simulink model "PrkgClimaActvMgr"  (R2022b)
settings: Solver=FixedStepDiscrete  StartTime=0.0  StopTime=10.0  FixedStep=0.2  RelTol=1e-3
(part 3 of 4)

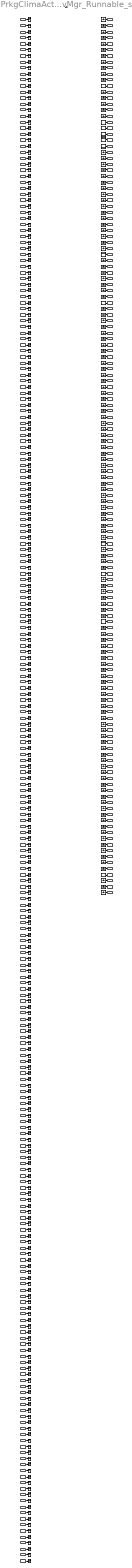
== system PrkgClimaActvMgr/PrkgClimaActvMgr_Runnable_sys ==
  ActvRqrdForPostPrkgClima_ActvRqrdForPostPrkgClima_read : Inport
  CarTiIntForClima_CarTiIntForClima_read : Inport  Port=2
  CdsClimaOnReqfromExsrc_CdsClimaOnReqfromExsrc_read : Inport  Port=3
  ClimaCfgForClimaCtrlMan1_ClimaCfgForClimaCtrlMan1_read : Inport  Port=4
  ClimaCfgForCmptmtClng_ClimaCfgForCmptmtClng_read : Inport  Port=5
  ClimaCfgForFoldCSD_ClimaCfgForFoldCSD_read : Inport  Port=6
  ClimaCfgForNrOfTSetg1_ClimaCfgForNrOfTSetg1_read : Inport  Port=7
  ClimaCfgForPreClng1_ClimaCfgForPreClng1_read : Inport  Port=8
  ClimaCfgForSeatHeatgForRowFirstPrsnt1_ClimaCfgForSeatHeatgForRowFirstPrsnt1_read : Inport  Port=9
  ClimaCfgForSeatHeatgForRowSec_ClimaCfgForSeatHeatgForRowSec_read : Inport  Port=10
  ClimaCfgForSeatVentnForRowFirst_ClimaCfgForSeatVentnForRowFirst_read : Inport  Port=11
  ClimaCfgForSeatVentnForRowSec_ClimaCfgForSeatVentnForRowSec_read : Inport  Port=12
  ClimaCfgForSteerWhlHeatgPrsnt1_ClimaCfgForSteerWhlHeatgPrsnt1_read : Inport  Port=13
  ClimaCfgForSteerWhlLeSide1_ClimaCfgForSteerWhlLeSide1_read : Inport  Port=14
  ClimaCfgForVehElec1_ClimaCfgForVehElec1_read : Inport  Port=15
  ClimaCfgForVehHyb1_ClimaCfgForVehHyb1_read : Inport  Port=16
  ClimaCfgForWindHeatrPrsnt1_ClimaCfgForWindHeatrPrsnt1_read : Inport  Port=17
  ClimaOvrHeatProWarnIni_ClimaOvrHeatProWarnIni_read : Inport  Port=18
  ClimaOvrHeatReqFromExrsc_ClimaOvrHeatReqFromExrsc_read : Inport  Port=19
  ClimaOvrHeatStrtReq_ClimaOvrHeatStrtReq_read : Inport  Port=20
  CmptmtClsd_CmptmtClsd_read : Inport  Port=21
  CSDUsgChgeOffReq_CSDUsgChgeOffReq_read : Inport  Port=22
  CSDUsgChgeOnReq_CSDUsgChgeOnReq_read : Inport  Port=23
  DefrstHeatgForPrkgClimaEnad_DefrstHeatgForPrkgClimaEnad_read : Inport  Port=24
  DoorOpenStsForClima_DoorOpenStsForClima_read : Inport  Port=25
  EgyAllwdForPrkgClima_EgyAllwdForPrkgClima_read : Inport  Port=26
  EgyPrioForPrkgClima_EgyPrioForPrkgClima_read : Inport  Port=27
  ErsCmdByKeyStrtReq_ErsCmdByKeyStrtReq_read : Inport  Port=28
  ErsRunTimelStrtReq_ErsRunTimelStrtReq_read : Inport  Port=29
  FacForRtForPrkgClima_FacForRtForPrkgClima_read : Inport  Port=30
  FrntHvacBlowerSts_FrntHvacBlowerSts_read : Inport  Port=31
  HeatrTypForPrkgClima_HeatrTypForPrkgClima_read : Inport  Port=32
  HeatUpRqrdForPrkgClima_HeatUpRqrdForPrkgClima_read : Inport  Port=33
  HMIExtVentFanVolReqStrtReq_HMIExtVentFanVolReqStrtReq_read : Inport  Port=34
  HMIExtVentonoffReqStrtReq_HMIExtVentonoffReqStrtReq_read : Inport  Port=35
  HMIExtVentTSpReqStrtReq_HMIExtVentTSpReqStrtReq_read : Inport  Port=36
  HMIExtVentTStsReqStrtReq_HMIExtVentTStsReqStrtReq_read : Inport  Port=37
  HvBattForPrkgClima_HvBattForPrkgClima_read : Inport  Port=38
  HvBattSoc_HvBattSoc_read : Inport  Port=39
  HvSysCnctWoU_HvSysCnctWoU_read : Inport  Port=40
  HvSysWoU_HvSysWoU_read : Inport  Port=41
  HvSysRlySts_HvSysRlySts_read : Inport  Port=42
  IntCo2MontrStrtReq_IntCo2MontrStrtReq_read : Inport  Port=43
  LockgCenSts_Ngcc_LockgCenSts_read : Inport  Port=44
  MemReadForPrkgClimaActvMgr_MemReadForPrkgClimaActvMgr_read : Inport  Port=45
  MemReqStsForPrkgClima_MemReqStsForPrkgClima_read : Inport  Port=46
  OffForTLoForPostCdng_OffForTLoForPostCdng_read : Inport  Port=47
  OffRqrdForPrkgClima_OffRqrdForPrkgClima_read : Inport  Port=48
  PrePrkgClimaNotAvlForHvSysWoU_PrePrkgClimaNotAvlForHvSysWoU_read : Inport  Port=49
  PrkgCDSAvl_PrkgCDSAvl_read : Inport  Port=50
  PrkgClimaAllwdForBattULo_PrkgClimaAllwdForBattULo_read : Inport  Port=51
  PrkgClimaAllwdForSwtHeatrTyp_PrkgClimaAllwdForSwtHeatrTyp_read : Inport  Port=52
  PrkgHeatgActv_PrkgHeatgActv_read : Inport  Port=53
  PwrAllwdForHvacFanFrnt_PwrAllwdForHvacFanFrnt_read : Inport  Port=54
  PwrCnsDesForHvacFanFrnt_PwrCnsDesForHvacFanFrnt_read : Inport  Port=55
  RemClimaallExsrcReq_RemClimaallExsrcReq_read : Inport  Port=56
  RemClimaEgyExsrcReq_RemClimaEgyExsrcReq_read : Inport  Port=57
  RemClimaTmrStrtReq_RemClimaTmrStrtReq_read : Inport  Port=58
  RemStrtExtnTiStrtReq_RemStrtExtnTiStrtReq_read : Inport  Port=59
  RemStrtHvCtrlStrtReq_RemStrtHvCtrlStrtReq_read : Inport  Port=60
  RemVentReqStrtReq_RemVentReqStrtReq_read : Inport  Port=61
  RtCmftForImdtPrePrkgClima_RtCmftForImdtPrePrkgClima_read : Inport  Port=62
  RtCmftForPostPrkgClima_RtCmftForPostPrkgClima_read : Inport  Port=63
  RtCmftForPreClng_RtCmftForPreClng_read : Inport  Port=64
  RtSdlForPrkgClima_RtSdlForPrkgClima_read : Inport  Port=65
  SeatHeatForPrkgClimaEnad_SeatHeatForPrkgClimaEnad_read : Inport  Port=66
  SteerWhlHeatgForPrkgClimaEnad_SteerWhlHeatgForPrkgClimaEnad_read : Inport  Port=67
  StrtRemSts1WdStrtRemSts_Ngcc_StrtRemSts1WdStrtRemSts_read : Inport  Port=68
  StrtRqrdForDirForPrePrkgClima_StrtRqrdForDirForPrePrkgClima_read : Inport  Port=69
  StrtRqrdForPreClng_StrtRqrdForPreClng_read : Inport  Port=70
  StrtRqrdForTmrForPrePrkgClima_StrtRqrdForTmrForPrePrkgClima_read : Inport  Port=71
  SuspStrtAllwdForPrkgClima_SuspStrtAllwdForPrkgClima_read : Inport  Port=72
  TelmBookClimaReqHmiCmptmtTSpSpclStrtReq_TelmBookClimaReqHmiCmptmtTSpSpclStrtReq_read : Inport  Port=73
  TelmBookClimaReqOnOffNoReqStrtReq_TelmBookClimaReqOnOffNoReqStrtReq_read : Inport  Port=74
  TelmBookClimaReqTempRangeStrtReq_TelmBookClimaReqTempRangeStrtReq_read : Inport  Port=75
  TelmBookClimaTmrStrtReq_TelmBookClimaTmrStrtReq_read : Inport  Port=76
  TelmBookSeatHeatClimalReqDrvHeatClimaLvlStrtReq_TelmBookSeatHeatClimalReqDrvHeatClimaLvlStrtReq_read : Inport  Port=77
  TelmBookSeatHeatClimalReqPassHeatClimaLvlStrtReq_TelmBookSeatHeatClimalReqPassHeatClimaLvlStrtReq_read : Inport  Port=78
  TelmBookSeatHeatClimalReqSecLeHeatClimaLvlStrtReq_TelmBookSeatHeatClimalReqSecLeHeatClimaLvlStrtReq_read : Inport  Port=79
  TelmBookSeatHeatClimalReqSecRiHeatClimaLvlStrtReq_TelmBookSeatHeatClimalReqSecRiHeatClimaLvlStrtReq_read : Inport  Port=80
  TelmBookSeatHeatClimalReqThrdLeHeatClimaLvlStrtReq_TelmBookSeatHeatClimalReqThrdLeHeatClimaLvlStrtReq_read : Inport  Port=81
  TelmBookSeatHeatClimalReqThrdRiHeatClimaLvlStrtReq_TelmBookSeatHeatClimalReqThrdRiHeatClimaLvlStrtReq_read : Inport  Port=82
  TelmBookSeatVentnClimalReqDrvVentnClimaLvlStrtReq_TelmBookSeatVentnClimalReqDrvVentnClimaLvlStrtReq_read : Inport  Port=83
  TelmBookSeatVentnClimalReqPassVentnClimaLvlStrtReq_TelmBookSeatVentnClimalReqPassVentnClimaLvlStrtReq_read : Inport  Port=84
  TelmBookSeatVentnClimalReqSecLeVentnClimaLvlStrtReq_TelmBookSeatVentnClimalReqSecLeVentnClimaLvlStrtReq_read : Inport  Port=85
  TelmBookSeatVentnClimalReqSecRiVentnClimaLvlStrtReq_TelmBookSeatVentnClimalReqSecRiVentnClimaLvlStrtReq_read : Inport  Port=86
  TelmBookSeatVentnClimalReqThrdLeVentnClimaLvlStrtReq_TelmBookSeatVentnClimalReqThrdLeVentnClimaLvlStrtReq_read : Inport  Port=87
  TelmBookSeatVentnClimalReqThrdRiVentnClimaLvlStrtReq_TelmBookSeatVentnClimalReqThrdRiVentnClimaLvlStrtReq_read : Inport  Port=88
  TelmBookSteerWhlHeatClimalReqStrtReq_TelmBookSteerWhlHeatClimalReqStrtReq_read : Inport  Port=89
  TelmClimaExtVentnLvlStrtReq_TelmClimaExtVentnLvlStrtReq_read : Inport  Port=90
  TelmClimaExtVentnReqStrtReq_TelmClimaExtVentnReqStrtReq_read : Inport  Port=91
  TelmClimaExtVentnTempRangeStrtReq_TelmClimaExtVentnTempRangeStrtReq_read : Inport  Port=92
  TelmClimaExtVentnTSpSpclStrtReq_TelmClimaExtVentnTSpSpclStrtReq_read : Inport  Port=93
  UpdRtTotForTmrForPrePrkgClima_UpdRtTotForTmrForPrePrkgClima_read : Inport  Port=94
  VehModMngtGlbSafe1_Ngcc_VehModMngtGlbSafe1_read : Inport  Port=95
  VFCBodyPreClimatisationHmiHumCtrl_VFCBodyPreClimatisationHmiHumCtrl_read : Inport  Port=96
  VFCHmiClimaCtrlAct_VFCHmiClimaCtrlAct_read : Inport  Port=97
  VFCPrkDrvgClimabyFragAct_VFCPrkDrvgClimabyFragAct_read : Inport  Port=98
  WarnForEgyAllwdLimd_WarnForEgyAllwdLimd_read : Inport  Port=99
  WarnForHybWoU_WarnForHybWoU_read : Inport  Port=100
  WarnForPreCdngFctNotAvl_WarnForPreCdngFctNotAvl_read : Inport  Port=101
  WarnForPrkgClimaNotAllwd_WarnForPrkgClimaNotAllwd_read : Inport  Port=102
  WindIceDetdFrnt_Ngcc_WindIceDetdFrnt_read : Inport  Port=103
  WindIceDetdRe_Ngcc_WindIceDetdRe_read : Inport  Port=104
  AirCdnAvl_AirCdnAvl_read : Inport  Port=105
  AmbTFildForClimaNgcc_AmbTFildForClima_read : Inport  Port=106
  OffRqrdForRemClima_OffRqrdForRemClima_read : Inport  Port=107
  RemClimaTempStrtReq_RemClimaTempStrtReq_read : Inport  Port=108
  VFCAutDemstOperSts_VFCAutDemstOperSts_read : Inport  Port=109
  VFCPrkDrvgClimabyPM25_VFCPrkDrvgClimabyPM25_read : Inport  Port=110
  AirCdnAvlRe_AirCdnAvlRe_read : Inport  Port=111
  AirSrcStsFbNgcc_AirSrcStsFbNgcc_read : Inport  Port=112
  BookTrvlClimaActvReqfromExsrc_BookTrvlClimaActvReqfromExsrc_read : Inport  Port=113
  CCMWakeupFunction_CCMWakeupFunction_read : Inport  Port=114
  ClimaCfgFor3RDROWSEATHEAT_ClimaCfgFor3RDROWSEATHEAT_read : Inport  Port=115
  ClimaCfgFor3RDROWSEATVENTILATED_ClimaCfgFor3RDROWSEATVENTILATED_read : Inport  Port=116
  ClimaCfgForAirMtrlSnsrPrsnt1_ClimaCfgForAirMtrlSnsrPrsnt1_read : Inport  Port=117
  ClimaCfgForBRAND_ClimaCfgForBRAND_read : Inport  Port=118
  ClimaCfgForDrvrSeatVentWthSmf2_ClimaCfgForDrvrSeatVentWthSmf2_read : Inport  Port=119
  ClimaCfgForErev1_ClimaCfgForErev1_read : Inport  Port=120
  ClimaCfgForHvacRePrsnt1_ClimaCfgForHvacRePrsnt1_read : Inport  Port=121
  ClimaCfgForHvacTyp_ClimaCfgForHvacTyp_read : Inport  Port=122
  ClimaCfgForLifeDetection_ClimaCfgForLifeDetection_read : Inport  Port=123
  ClimaCfgForMARKET_ClimaCfgForMARKET_read : Inport  Port=124
  ClimaCfgForPassSeatVentWthSmf2_ClimaCfgForPassSeatVentWthSmf2_read : Inport  Port=125
  ClimaCfgForPrpsnTyp_ClimaCfgForPrpsnTyp_read : Inport  Port=126
  ClimaCfgForRoofHatch_ClimaCfgForRoofHatch_read : Inport  Port=127
  ClimaCfgForVehTyp_ClimaCfgForVehTyp_read : Inport  Port=128
  ClimaCfgReqVersionReplace_ClimaCfgReqVersionReplace_read : Inport  Port=129
  CmptmtClngActv_CmptmtClngActv_read : Inport  Port=130
  CmptmtOpenStsForClima_CmptmtOpenStsForClima_read : Inport  Port=131
  DoorStsWithOutTrForClima_DoorStsWithOutTrForClima_read : Inport  Port=132
  DrvrSeatHeatgLvlSts_DrvrSeatHeatgLvlSts_read : Inport  Port=133
  DrvrSeatHeatgLvlSts_DrvrSeatHeatgLvlSts_IsUpdated_read : Inport  Port=134
  DrvrSeatVentnLvlSts_DrvrSeatVentnLvlSts_read : Inport  Port=135
  DrvrSeatVentnLvlSts_DrvrSeatVentnLvlSts_IsUpdated_read : Inport  Port=136
  DrvrSeatVentnLvlSts2Ngcc_DrvrSeatVentnLvlSts2Ngcc_read : Inport  Port=137
  EngSt1WdSts_Ngcc_EngSt1WdSts_read : Inport  Port=138
  ErsCmdByKeyFromExsrc_ErsCmdByKeyFromExsrc_read : Inport  Port=139
  GearLvrIndcn_GearLvrIndcn_read : Inport  Port=140
  GearLvrIndcn_GearLvrIndcn_IsUpdated_read : Inport  Port=141
  HighTempCleanHmiReqNgcc_HighTempCleanHmiReqNgcc_read : Inport  Port=142
  HMIAirCdnrAutDrySetNgcc_HMIAirCdnrAutDrySetNgcc_read : Inport  Port=143
  HMIAirCdnrAutDrySetNgcc_HMIAirCdnrAutDrySetNgcc_IsUpdated_read : Inport  Port=144
  HmiCmptmtTSp_HmiCmptmtTSp_read : Inport  Port=145
  HMILBRACStrtSetNgcc_HMILBRACStrtSetNgcc_read : Inport  Port=146
  HmiParkVentSetNgcc_HmiParkVentSetNgcc_read : Inport  Port=147
  HmiParkVentTmrSetNgcc_HmiParkVentTmrSetNgcc_read : Inport  Port=148
  HvBattMntnActv_HvBattMntnActv_read : Inport  Port=149
  HvCooltHeatrStsSig_HvCooltHeatrStsSig_read : Inport  Port=150
  HvCooltHeatrStsSig_HvCooltHeatrStsSig_IsUpdated_read : Inport  Port=151
  IntPm25VluFrmClima_IntPm25VluFrmClima_read : Inport  Port=152
  LDACState_LDACState_read : Inport  Port=153
  MemBookTrvlClimaTi_MemBookTrvlClimaTi_read : Inport  Port=154
  MemReadForBlwAftRun_MemReadForBlwAftRun_read : Inport  Port=155
  MemReadForHighTempCln_MemReadForHighTempCln_read : Inport  Port=156
  MemReadForHMISetEngReq_MemReadForHMISetEngReq_read : Inport  Port=157
  MemReadForParkVent_MemReadForParkVent_read : Inport  Port=158
  MemReadForParkVentActvCnt_MemReadForParkVentActvCnt_read : Inport  Port=159
  OnBdChrgrSt_OnBdChrgrSt_read : Inport  Port=160
  OnBdChrgrSt_OnBdChrgrSt_IsUpdated_read : Inport  Port=161
  OxygenSplyAvlStsNgcc_OxygenSplyAvlStsNgcc_read : Inport  Port=162
  OxygenSplyErrNgcc_OxygenSplyErrNgcc_read : Inport  Port=163
  PassSeatHeatgLvlSts_PassSeatHeatgLvlSts_read : Inport  Port=164
  PassSeatHeatgLvlSts_PassSeatHeatgLvlSts_IsUpdated_read : Inport  Port=165
  PassSeatVentnLvlSts_PassSeatVentnLvlSts_read : Inport  Port=166
  PassSeatVentnLvlSts_PassSeatVentnLvlSts_IsUpdated_read : Inport  Port=167
  PassSeatVentnLvlSts2Ngcc_PassSeatVentnLvlSts2Ngcc_read : Inport  Port=168
  PrkgClimaReqExcpSeat_PrkgClimaReqExcpSeat_read : Inport  Port=169
  PrkgClimaReqPrio_PrkgClimaReqPrio_read : Inport  Port=170
  PrkgOGMAirSrcReq_PrkgOGMAirSrcReq_read : Inport  Port=171
  PrvntWinActvReq_PrvntWinActvReq_read : Inport  Port=172
  ReHvacBlowerSts_ReHvacBlowerSts_read : Inport  Port=173
  RemClimaEgyExsrcReqPlus_RemClimaEgyExsrcReqPlus_read : Inport  Port=174
  RemClimaHVReqFb_RemClimaHVReqFb_read : Inport  Port=175
  RemClimaHVReqFb_RemClimaHVReqFb_IsUpdated_read : Inport  Port=176
  RemClimaWarnForCmptmtClng_RemClimaWarnForCmptmtClng_read : Inport  Port=177
  RemClimaWarnForOpenGlb_RemClimaWarnForOpenGlb_read : Inport  Port=178
  RemClimaWarnForUnLckClima_RemClimaWarnForUnLckClima_read : Inport  Port=179
  RemOxygenSplyReq_RemOxygenSplyReq_read : Inport  Port=180
  SeatHeatgLvlStsRowSecLe_SeatHeatgLvlStsRowSecLe_read : Inport  Port=181
  SeatHeatgLvlStsRowSecLe_SeatHeatgLvlStsRowSecLe_IsUpdated_read : Inport  Port=182
  SeatHeatgLvlStsRowSecRi_SeatHeatgLvlStsRowSecRi_read : Inport  Port=183
  SeatHeatgLvlStsRowSecRi_SeatHeatgLvlStsRowSecRi_IsUpdated_read : Inport  Port=184
  SeatHeatgLvlStsRowThrdLe_SeatHeatgLvlStsRowThrdLe_read : Inport  Port=185
  SeatHeatgLvlStsRowThrdLe_SeatHeatgLvlStsRowThrdLe_IsUpdated_read : Inport  Port=186
  SeatHeatgLvlStsRowThrdRi_SeatHeatgLvlStsRowThrdRi_read : Inport  Port=187
  SeatHeatgLvlStsRowThrdRi_SeatHeatgLvlStsRowThrdRi_IsUpdated_read : Inport  Port=188
  SeatVentnLvlStsRowSecLe_SeatVentnLvlStsRowSecLe_read : Inport  Port=189
  SeatVentnLvlStsRowSecLe_SeatVentnLvlStsRowSecLe_IsUpdated_read : Inport  Port=190
  SeatVentnLvlStsRowSecRi_SeatVentnLvlStsRowSecRi_read : Inport  Port=191
  SeatVentnLvlStsRowSecRi_SeatVentnLvlStsRowSecRi_IsUpdated_read : Inport  Port=192
  SeatVentnLvlStsRowThrdLe_SeatVentnLvlStsRowThrdLe_read : Inport  Port=193
  SeatVentnLvlStsRowThrdLe_SeatVentnLvlStsRowThrdLe_IsUpdated_read : Inport  Port=194
  SeatVentnLvlStsRowThrdRi_SeatVentnLvlStsRowThrdRi_read : Inport  Port=195
  SeatVentnLvlStsRowThrdRi_SeatVentnLvlStsRowThrdRi_IsUpdated_read : Inport  Port=196
  SteerWhlHeatgDurgClimaEnadFromExsrc_SteerWhlHeatgDurgClimaEnadFromExsrc_read : Inport  Port=197
  SunRoofPosnSts_Ngcc_SunRoofPosnSts_read : Inport  Port=198
  TcsOvrheatdProtnReq_TcsOvrheatdProtnReq_read : Inport  Port=199
  TelmClimaTmrFromExtSrc_TelmClimaTmrFromExtSrc_read : Inport  Port=200
  TelmDefrostTmr_TelmDefrostTmr_read : Inport  Port=201
  TelmDefrostTmr_TelmDefrostTmr_IsUpdated_read : Inport  Port=202
  TelmOxygenReqFromExSrc_TelmOxygenReqFromExSrc_read : Inport  Port=203
  TelmOxygenTmr_TelmOxygenTmr_read : Inport  Port=204
  TelmOxygenTmr_TelmOxygenTmr_IsUpdated_read : Inport  Port=205
  TelmSeatDrvrHeatReqFromExsrc_TelmSeatDrvrHeatReqFromExsrc_read : Inport  Port=206
  TelmSeatDrvVentnReqFromExsrc_TelmSeatDrvVentnReqFromExsrc_read : Inport  Port=207
  TelmSeatHeatDrvrTmr_TelmSeatHeatDrvrTmr_read : Inport  Port=208
  TelmSeatHeatDrvrTmr_TelmSeatHeatDrvrTmr_IsUpdated_read : Inport  Port=209
  TelmSeatHeatPassTmr_TelmSeatHeatPassTmr_read : Inport  Port=210
  TelmSeatHeatPassTmr_TelmSeatHeatPassTmr_IsUpdated_read : Inport  Port=211
  TelmSeatHeatSecLeTmr_TelmSeatHeatSecLeTmr_read : Inport  Port=212
  TelmSeatHeatSecLeTmr_TelmSeatHeatSecLeTmr_IsUpdated_read : Inport  Port=213
  TelmSeatHeatSecRiTmr_TelmSeatHeatSecRiTmr_read : Inport  Port=214
  TelmSeatHeatSecRiTmr_TelmSeatHeatSecRiTmr_IsUpdated_read : Inport  Port=215
  TelmSeatHeatThrdLeTmr_TelmSeatHeatThrdLeTmr_read : Inport  Port=216
  TelmSeatHeatThrdLeTmr_TelmSeatHeatThrdLeTmr_IsUpdated_read : Inport  Port=217
  TelmSeatHeatThrdRiTmr_TelmSeatHeatThrdRiTmr_read : Inport  Port=218
  TelmSeatHeatThrdRiTmr_TelmSeatHeatThrdRiTmr_IsUpdated_read : Inport  Port=219
  TelmSeatPassHeatReqFromExsrc_TelmSeatPassHeatReqFromExsrc_read : Inport  Port=220
  TelmSeatPassVentnReqFromExsrc_TelmSeatPassVentnReqFromExsrc_read : Inport  Port=221
  TelmSeatSecLeHeatReqFromExsrc_TelmSeatSecLeHeatReqFromExsrc_read : Inport  Port=222
  TelmSeatSecLeVentnReqFromExsrc_TelmSeatSecLeVentnReqFromExsrc_read : Inport  Port=223
  TelmSeatSecRiHeatReqFromExsrc_TelmSeatSecRiHeatReqFromExsrc_read : Inport  Port=224
  TelmSeatSecRiVentnReqFromExsrc_TelmSeatSecRiVentnReqFromExsrc_read : Inport  Port=225
  TelmSeatThrdLeHeatReqFromExsrc_TelmSeatThrdLeHeatReqFromExsrc_read : Inport  Port=226
  TelmSeatThrdLeVentnReqFromExsrc_TelmSeatThrdLeVentnReqFromExsrc_read : Inport  Port=227
  TelmSeatThrdRiHeatReqFromExsrc_TelmSeatThrdRiHeatReqFromExsrc_read : Inport  Port=228
  TelmSeatThrdRiVentnReqFromExsrc_TelmSeatThrdRiVentnReqFromExsrc_read : Inport  Port=229
  TelmSeatVentnDrvrTmr_TelmSeatVentnDrvrTmr_read : Inport  Port=230
  TelmSeatVentnDrvrTmr_TelmSeatVentnDrvrTmr_IsUpdated_read : Inport  Port=231
  TelmSeatVentnPassTmr_TelmSeatVentnPassTmr_read : Inport  Port=232
  TelmSeatVentnPassTmr_TelmSeatVentnPassTmr_IsUpdated_read : Inport  Port=233
  TelmSeatVentnSecLeTmr_TelmSeatVentnSecLeTmr_read : Inport  Port=234
  TelmSeatVentnSecLeTmr_TelmSeatVentnSecLeTmr_IsUpdated_read : Inport  Port=235
  TelmSeatVentnSecRiTmr_TelmSeatVentnSecRiTmr_read : Inport  Port=236
  TelmSeatVentnSecRiTmr_TelmSeatVentnSecRiTmr_IsUpdated_read : Inport  Port=237
  TelmSeatVentnThrdLeTmr_TelmSeatVentnThrdLeTmr_read : Inport  Port=238
  TelmSeatVentnThrdLeTmr_TelmSeatVentnThrdLeTmr_IsUpdated_read : Inport  Port=239
  TelmSeatVentnThrdReq_TelmSeatVentnThrdReq_read : Inport  Port=240
  TelmSeatVentnThrdRiTmr_TelmSeatVentnThrdRiTmr_read : Inport  Port=241
  TelmSeatVentnThrdRiTmr_TelmSeatVentnThrdRiTmr_IsUpdated_read : Inport  Port=242
  TelmSteerWhlHeatTmr_TelmSteerWhlHeatTmr_read : Inport  Port=243
  TelmSteerWhlHeatTmr_TelmSteerWhlHeatTmr_IsUpdated_read : Inport  Port=244
  UnLckClimaActvReq_UnLckClimaActvReq_read : Inport  Port=245
  UnLckClimaActvReqSt_UnLckClimaActvReqSt_read : Inport  Port=246
  UnLckHeatActvReq_UnLckHeatActvReq_read : Inport  Port=247
  UnLckHeatActvReqSt_UnLckHeatActvReqSt_read : Inport  Port=248
  VentnForWinOpenGlbActv_VentnForWinOpenGlbActv_read : Inport  Port=249
  VFCPrkDrvgClimabyOxygenSplyCtrl_VFCPrkDrvgClimabyOxygenSplyCtrl_read : Inport  Port=250
  WarnLvlMsgNgcc_WarnLvlMsgNgcc_read : Inport  Port=251
  WarnLvlMsgNgcc_WarnLvlMsgNgcc_IsUpdated_read : Inport  Port=252
  WinOpenGlbReq_WinOpenGlbReq_read : Inport  Port=253
  WinPosnStsAtReLe_WinPosnStsAtReLe_read : Inport  Port=254
  WinPosnStsAtReRi_WinPosnStsAtReRi_read : Inport  Port=255
  WinPosnStsDrv_WinPosnStsAtDrv_read : Inport  Port=256
  WinPosnStsPass_WinPosnStsAtPass_read : Inport  Port=257
  function : TriggerPort  TriggerType=function-call  VariantControl=(inherit)  SampleTimeType=periodic  SampleTime=-1
  Ground_{1..11,13..17,23..39,41..47,49..87,89..92,94..100,102..142,144..146} (134 alike) : Ground
  Ground_101 : Subsystem
  Ground_12 : Subsystem
  Ground_143 : Subsystem
  Ground_18 : Subsystem
  Ground_19 : Subsystem
  Ground_20 : Subsystem
  Ground_21 : Subsystem
  Ground_22 : Subsystem
  Ground_40 : Subsystem
  Ground_48 : Subsystem
  Ground_88 : Subsystem
  Ground_93 : Subsystem
  Terminator_{1..257} (257 alike) : Terminator
  BlwAftRunAct_BlwAftRunAct_write : Outport
      settings: EnsureOutportIsVirtual=on
  BlwAftRunReqRe_BlwAftRunReqRe_write : Outport  Port=2
      settings: EnsureOutportIsVirtual=on
  BlwAftRunWkTime_BlwAftRunWkTime_write : Outport  Port=3
      settings: EnsureOutportIsVirtual=on
  BookTrvlClimaFnshd_BookTrvlClimaFnshd_write : Outport  Port=4
      settings: EnsureOutportIsVirtual=on
  DsChrgClima_DsChrgClima_write : Outport  Port=5
      settings: EnsureOutportIsVirtual=on
  HghClnAct_HghClnAct_write : Outport  Port=6
      settings: EnsureOutportIsVirtual=on
  HghClnWkTime_HghClnWkTime_write : Outport  Port=7
      settings: EnsureOutportIsVirtual=on
  HighTempCleanRunningStsNgcc_HighTempCleanRunningStsNgcc_write : Outport  Port=8
      settings: EnsureOutportIsVirtual=on
  HighTempCleanSettingStsNgcc_HighTempCleanSettingStsNgcc_write : Outport  Port=9
      settings: EnsureOutportIsVirtual=on
  HighTempClnActv_HighTempClnActv_write : Outport  Port=10
      settings: EnsureOutportIsVirtual=on
  KeyClimaActvFnshd_KeyClimaActvFnshd_write : Outport  Port=11
      settings: EnsureOutportIsVirtual=on
  KeySynchrnztnLclNgcc_KeySynchrnztnLclNgcc_write : Outport  Port=12
      settings: EnsureOutportIsVirtual=on
  MemSaveFlgForBlwAftRun_MemSaveFlgForBlwAftRun_write : Outport  Port=13
      settings: EnsureOutportIsVirtual=on
  MemSaveFlgForHighTempCln_MemSaveFlgForHighTempCln_write : Outport  Port=14
      settings: EnsureOutportIsVirtual=on
  MemSaveFlgForHMISetEngReq_MemSaveFlgForHMISetEngReq_write : Outport  Port=15
      settings: EnsureOutportIsVirtual=on
  MemSaveFlgForParkVent_MemSaveFlgForParkVent_write : Outport  Port=16
      settings: EnsureOutportIsVirtual=on
  MemSaveFlgForParkVentActvCnt_MemSaveFlgForParkVentActvCnt_write : Outport  Port=17
      settings: EnsureOutportIsVirtual=on
  MemWrForBlwAftRun_MemWrForBlwAftRun_write : Outport  Port=18
      settings: EnsureOutportIsVirtual=on
  MemWrForHighTempCln_MemWrForHighTempCln_write : Outport  Port=19
      settings: EnsureOutportIsVirtual=on
  MemWrForHMISetEngReq_MemWrForHMISetEngReq_write : Outport  Port=20
      settings: EnsureOutportIsVirtual=on
  MemWrForParkVent_MemWrForParkVent_write : Outport  Port=21
      settings: EnsureOutportIsVirtual=on
  MemWrForParkVentActvCnt_MemWrForParkVentActvCnt_write : Outport  Port=22
      settings: EnsureOutportIsVirtual=on
  OGMAirSrcFnshd_OGMAirSrcFnshd_write : Outport  Port=23
      settings: EnsureOutportIsVirtual=on
  OGMAirSrcReq_OGMAirSrcReq_write : Outport  Port=24
      settings: EnsureOutportIsVirtual=on
  OGMAvlSts_OGMAvlSts_write : Outport  Port=25
      settings: EnsureOutportIsVirtual=on
  OGMReq_OGMReq_write : Outport  Port=26
      settings: EnsureOutportIsVirtual=on
  ParkVentActv_ParkVentActv_write : Outport  Port=27
      settings: EnsureOutportIsVirtual=on
  ParkVentWkTi_ParkVentWkTi_write : Outport  Port=28
      settings: EnsureOutportIsVirtual=on
  PrkgClimaACReq_PrkgClimaACReq_write : Outport  Port=29
      settings: EnsureOutportIsVirtual=on
  PrkgClimaActvMgrVFCLDACClimaCtrl_PrkgClimaActvMgrVFCLDACClimaCtrl_write : Outport  Port=30
      settings: EnsureOutportIsVirtual=on
  PrkgClimaAirflowReqRe_PrkgClimaAirflowReqRe_write : Outport  Port=31
      settings: EnsureOutportIsVirtual=on
  PrkgClimaEngStReqNgcc_PrkgClimaEngStReqNgcc_write : Outport  Port=32
      settings: EnsureOutportIsVirtual=on
  PrkgClimaHVReq_PrkgClimaHVReq_write : Outport  Port=33
      settings: EnsureOutportIsVirtual=on
  PrkgClimaOnReqRe_PrkgClimaOnReqRe_write : Outport  Port=34
      settings: EnsureOutportIsVirtual=on
  PrkgCmftRlyReq_PrkgCmftRlyReq_write : Outport  Port=35
      settings: EnsureOutportIsVirtual=on
  PrkgOGMAirSrcActv_PrkgOGMAirSrcActv_write : Outport  Port=36
      settings: EnsureOutportIsVirtual=on
  PrkgVntFctn_PrkgVntFctn_write : Outport  Port=37
      settings: EnsureOutportIsVirtual=on
  PrvntWinFrz_PrvntWinFrz_write : Outport  Port=38
      settings: EnsureOutportIsVirtual=on
  PrvntWinFrzFnshd_PrvntWinFrzFnshd_write : Outport  Port=39
      settings: EnsureOutportIsVirtual=on
  RemActvSts_RemActvSts_write : Outport  Port=40
      settings: EnsureOutportIsVirtual=on
  RemClimaFnshd1_RemClimaFnshd1_write : Outport  Port=41
      settings: EnsureOutportIsVirtual=on
  RemClimaWarn_RemClimaWarn_write : Outport  Port=42
      settings: EnsureOutportIsVirtual=on
  RemOxygenSplyActv_RemOxygenSplyActv_write : Outport  Port=43
      settings: EnsureOutportIsVirtual=on
  RemOxygenSplyActvFaildNgcc_RemOxygenSplyActvFaildNgcc_write : Outport  Port=44
      settings: EnsureOutportIsVirtual=on
  RemOxygenSplyFnshd_RemOxygenSplyFnshd_write : Outport  Port=45
      settings: EnsureOutportIsVirtual=on
  RemOxygenSplyFnshd1_RemOxygenSplyFnshd1_write : Outport  Port=46
      settings: EnsureOutportIsVirtual=on
  RemOxygenSplyWarn_RemOxygenSplyWarn_write : Outport  Port=47
      settings: EnsureOutportIsVirtual=on
  RemSynchrnztnLcl_RemSynchrnztnLcl_write : Outport  Port=48
      settings: EnsureOutportIsVirtual=on
  RemWinClsReq_RemWinClsReq_write : Outport  Port=49
      settings: EnsureOutportIsVirtual=on
  SeatClimaDurgClimaHmiSeatHeatgForRowThrdLe_SeatClimaDurgClimaHmiSeatHeatgForRowThrdLe_write : Outport  Port=50
      settings: EnsureOutportIsVirtual=on
  SeatClimaDurgClimaHmiSeatHeatgForRowThrdRi_SeatClimaDurgClimaHmiSeatHeatgForRowThrdRi_write : Outport  Port=51
      settings: EnsureOutportIsVirtual=on
  SeatClimaDurgClimaHmiSeatVentnForRowThrdLe_SeatClimaDurgClimaHmiSeatVentnForRowThrdLe_write : Outport  Port=52
      settings: EnsureOutportIsVirtual=on
  SeatClimaDurgClimaHmiSeatVentnForRowThrdRi_SeatClimaDurgClimaHmiSeatVentnForRowThrdRi_write : Outport  Port=53
      settings: EnsureOutportIsVirtual=on
  TelmDefrostFnshd_TelmDefrostFnshd_write : Outport  Port=54
      settings: EnsureOutportIsVirtual=on
  TelmSeatHeatDrvrFnshd_TelmSeatHeatDrvrFnshd_write : Outport  Port=55
      settings: EnsureOutportIsVirtual=on
  TelmSeatHeatPassFnshd_TelmSeatHeatPassFnshd_write : Outport  Port=56
      settings: EnsureOutportIsVirtual=on
  TelmSeatHeatSecLeFnshd_TelmSeatHeatSecLeFnshd_write : Outport  Port=57
      settings: EnsureOutportIsVirtual=on
  TelmSeatHeatSecRiFnshd_TelmSeatHeatSecRiFnshd_write : Outport  Port=58
      settings: EnsureOutportIsVirtual=on
  TelmSeatHeatThrdLeFnshd_TelmSeatHeatThrdLeFnshd_write : Outport  Port=59
      settings: EnsureOutportIsVirtual=on
  TelmSeatHeatThrdRiFnshd_TelmSeatHeatThrdRiFnshd_write : Outport  Port=60
      settings: EnsureOutportIsVirtual=on
  TelmSeatVentnDrvrFnshd_TelmSeatVentnDrvrFnshd_write : Outport  Port=61
      settings: EnsureOutportIsVirtual=on
  TelmSeatVentnPassFnshd_TelmSeatVentnPassFnshd_write : Outport  Port=62
      settings: EnsureOutportIsVirtual=on
  TelmSeatVentnSecLeFnshd_TelmSeatVentnSecLeFnshd_write : Outport  Port=63
      settings: EnsureOutportIsVirtual=on
  TelmSeatVentnSecRiFnshd_TelmSeatVentnSecRiFnshd_write : Outport  Port=64
      settings: EnsureOutportIsVirtual=on
  TelmSeatVentnThrdLeFnshd_TelmSeatVentnThrdLeFnshd_write : Outport  Port=65
      settings: EnsureOutportIsVirtual=on
  TelmSeatVentnThrdRiFnshd_TelmSeatVentnThrdRiFnshd_write : Outport  Port=66
      settings: EnsureOutportIsVirtual=on
  TelmSteerWhlHeatFnshd_TelmSteerWhlHeatFnshd_write : Outport  Port=67
      settings: EnsureOutportIsVirtual=on
  VMMChngNdMtnNgcc_VMMChngNdMtnNgcc_write : Outport  Port=68
      settings: EnsureOutportIsVirtual=on
  VTMOverTProtection_VTMOverTProtection_write : Outport  Port=69
      settings: EnsureOutportIsVirtual=on
  VTMOverTProtectionFnshd_VTMOverTProtectionFnshd_write : Outport  Port=70
      settings: EnsureOutportIsVirtual=on
  BlwAftRunReq_BlwAftRunReq_write : Outport  Port=71
      settings: EnsureOutportIsVirtual=on
  BlwAftRunSts_BlwAftRunSts_write : Outport  Port=72
      settings: EnsureOutportIsVirtual=on
  BookTrvlClimaActv_BookTrvlClimaActv_write : Outport  Port=73
      settings: EnsureOutportIsVirtual=on
  CdsParkgClimaActv_CdsParkgClimaActv_write : Outport  Port=74
      settings: EnsureOutportIsVirtual=on
  ClimaActv_Ngcc_ClimaActv_write : Outport  Port=75
      settings: EnsureOutportIsVirtual=on
  ClimaOvrHeatProActv_ClimaOvrHeatProActv_write : Outport  Port=76
      settings: EnsureOutportIsVirtual=on
  ClimaOvrHeatProActvSts_ClimaOvrHeatProActvSts_write : Outport  Port=77
      settings: EnsureOutportIsVirtual=on
  ClimaOvrHeatProWarn_ClimaOvrHeatProWarn_write : Outport  Port=78
      settings: EnsureOutportIsVirtual=on
  ClimaOvrHeatPrtSts_ClimaOvrHeatPrtSts_write : Outport  Port=79
      settings: EnsureOutportIsVirtual=on
  ClimaSts_ClimaSts_write : Outport  Port=80
      settings: EnsureOutportIsVirtual=on
  ClimaTiOfEnd_Ngcc_ClimaTiOfEnd_write : Outport  Port=81
      settings: EnsureOutportIsVirtual=on
  ClimaWarn_ClimaWarn_write : Outport  Port=82
      settings: EnsureOutportIsVirtual=on
  ClngActv_ClngActv_write : Outport  Port=83
      settings: EnsureOutportIsVirtual=on
  Co2WarnForTelm_Co2WarnForTelm_write : Outport  Port=84
      settings: EnsureOutportIsVirtual=on
  COHSts_COHSts_write : Outport  Port=85
      settings: EnsureOutportIsVirtual=on
  CoolgFastForPrePrkgClimaActv_CoolgFastForPrePrkgClimaActv_write : Outport  Port=86
      settings: EnsureOutportIsVirtual=on
  CoolgFastUniqueForPrePrkgClimaActv_CoolgFastUniqueForPrePrkgClimaActv_write : Outport  Port=87
      settings: EnsureOutportIsVirtual=on
  DefrstReq_DefrstReq_write : Outport  Port=88
      settings: EnsureOutportIsVirtual=on
  DiDblLock_DiDblLock_write : Outport  Port=89
      settings: EnsureOutportIsVirtual=on
  EcuKeepActvFromPrkgClima_EcuKeepActvFromPrkgClima_write : Outport  Port=90
      settings: EnsureOutportIsVirtual=on
  ExtVentFanVolStsFrmClima_ExtVentFanVolStsFrmClima_write : Outport  Port=91
      settings: EnsureOutportIsVirtual=on
  ExtVentStsFrmClima_ExtVentStsFrmClima_write : Outport  Port=92
      settings: EnsureOutportIsVirtual=on
  ExtVentTempStsFrmClima_ExtVentTempStsFrmClima_write : Outport  Port=93
      settings: EnsureOutportIsVirtual=on
  ExtVentTempStsFrmClimaExtVentTemprSts_ExtVentTempStsFrmClimaExtVentTemprSts_write : Outport  Port=94
      settings: EnsureOutportIsVirtual=on
  ExtVentTempStsFrmClimaExtVentTemprVal_ExtVentTempStsFrmClimaExtVentTemprVal_write : Outport  Port=95
      settings: EnsureOutportIsVirtual=on
  ExtVentToastFrmClima_Prkg_ExtVentToastFrmClima_write : Outport  Port=96
      settings: EnsureOutportIsVirtual=on
  HeatrElecFrntReqFromPrkgClima_HeatrElecFrntReqFromPrkgClima_write : Outport  Port=97
      settings: EnsureOutportIsVirtual=on
  IntCo2ActvSts_IntCo2ActvSts_write : Outport  Port=98
      settings: EnsureOutportIsVirtual=on
  KeyClimaActv_KeyClimaActv_write : Outport  Port=99
      settings: EnsureOutportIsVirtual=on
  MemSaveFlgForPrkgClimaActvMgr_MemSaveFlgForPrkgClimaActvMgr_write : Outport  Port=100
      settings: EnsureOutportIsVirtual=on
  MemWrForPrkgClimaActvMgr_MemWrForPrkgClimaActvMgr_write : Outport  Port=101
      settings: EnsureOutportIsVirtual=on
  ModForPrkgClima_ModForPrkgClima_write : Outport  Port=102
      settings: EnsureOutportIsVirtual=on
  PreClngActv_PreClngActv_write : Outport  Port=103
      settings: EnsureOutportIsVirtual=on
  PreClngNotif_PreClngNotif_write : Outport  Port=104
      settings: EnsureOutportIsVirtual=on
  PreClngSts_PreClngSts_write : Outport  Port=105
      settings: EnsureOutportIsVirtual=on
  PrkgClimaActvMgrVFCBodyPreClimatisation_PrkgClimaActvMgrVFCBodyPreClimatisation_write : Outport  Port=106
      settings: EnsureOutportIsVirtual=on
  PrkgClimaActvMgrVFCGlobalShortDuration_PrkgClimaActvMgrVFCGlobalShortDuration_write : Outport  Port=107
      settings: EnsureOutportIsVirtual=on
  PrkgClimaActvMgrVFCLocking_PrkgClimaActvMgrVFCLocking_write : Outport  Port=108
      settings: EnsureOutportIsVirtual=on
  PrkgClimaActvMgrVFCParkingDrivingClimatization_PrkgClimaActvMgrVFCParkingDrivingClimatization_write : Outport  Port=109
      settings: EnsureOutportIsVirtual=on
  PrkgClimaActvMgrVFCPostClimatisation_PrkgClimaActvMgrVFCPostClimatisation_write : Outport  Port=110
      settings: EnsureOutportIsVirtual=on
  PrkgClimaActvMgrVFCWindows_PrkgClimaActvMgrVFCWindows_write : Outport  Port=111
      settings: EnsureOutportIsVirtual=on
  PrkgClimaAirflowReq_PrkgClimaAirflowReq_write : Outport  Port=112
      settings: EnsureOutportIsVirtual=on
  PrkgClimaFnshd_PrkgClimaFnshd_write : Outport  Port=113
      settings: EnsureOutportIsVirtual=on
  PrkgClimaHeatgReq_PrkgClimaHeatgReq_write : Outport  Port=114
      settings: EnsureOutportIsVirtual=on
  PrkgClimaModReq_PrkgClimaModReq_write : Outport  Port=115